simulink model "Complete_Model"  (R2023a)
settings: Solver=VariableStepAuto  StartTime=0.0  StopTime=22  MinStep=5e-5  RelTol=1e-3
values from neighbouring files (symbols this model uses):
  Cbus = 80e-3*n_wt_agg*u^2    (= 1.33333333333)    [param.m]
  D = 1e-3    (= 0.001)    [param.m]
  f = 50    [param.m]
  Fs = Vs * sqrt(2/3)/(2*pi*f)    [param.m]
  fsw = 4e3    (= 4000)    [param.m]
  Idr_rated = sqrt(2)*Is    [param.m]
  J = 127*n_wt_agg    (= 19050)    [param.m]
  ki_id = (wni^2)*Lr*sigma    (= 3.27733001332)    [param.m]
  ki_idg = (wnig^2)*Lg    (= 0.263189450696)    [param.m]
  ki_iq = ki_id    (= 3.27733001332)    [param.m]
  ki_iqg = ki_idg    (= 0.263189450696)    [param.m]
  ki_v = -300000*n_wt_agg    (= -45000000)    [param.m]
  Kopt = ((0.5*rho*pi*(Radius^5)*Cp_max)/(lambda_opt^3))    (= 296454.198186)    [param.m]
  kp_id = (2*wni*sigma*Lr) - Rr    (= 0.00384733333333)    [param.m]
  kp_idg = ((2*wnig*Lg)-Rg)    (= 0.00167538274858)    [param.m]
  kp_iq = kp_id    (= 0.00384733333333)    [param.m]
  kp_iqg = kp_idg    (= 0.00167538274858)    [param.m]
  kp_v = -1000*n_wt_agg    (= -150000)    [param.m]
  Kpg = 1/(1.5*Vr*sqrt(2/3))    [param.m]
  Kqg = -Kpg    [param.m]
  lambda_opt = 7.2    [param.m]
  Lg = 400e-6 /n_wt_agg    (= 2.66666666667e-06)    [param.m]
  Lm = 2.5e-3 /n_wt_agg    (= 1.66666666667e-05)    [param.m]
  Lr = Lm + Lsi    (= 1.72466666667e-05)    [param.m]
  Ls = Lm + Lsi    (= 1.72466666667e-05)    [param.m]
  Lsi = 0.087e-3 /n_wt_agg    (= 5.8e-07)    [param.m]
  N = 100    [param.m]
  n_wt_agg = 150    [param.m]
  p = 2    [param.m]
  Ps = 2e6*n_wt_agg    (= 300000000)    [param.m]
  Radius = 42    [param.m]
  Rg = 20e-6 /n_wt_agg    (= 1.33333333333e-07)    [param.m]
  rho = 1.225    [param.m]
  Rr = 2.9e-3 /n_wt_agg    (= 1.93333333333e-05)    [param.m]
  Rs = 2.6e-3 /n_wt_agg    (= 1.73333333333e-05)    [param.m]
  sigma = 1 - Lm^2 / (Ls*Lr)    (= 0.0661284179513)    [param.m]
  smax = 1/3    (= 0.333333333333)    [param.m]
  tab_lambda = [0.1:0.01:11.8]    [param.m]
  Vbus = 1150/u    (= 3450)    [param.m]
  Vs = 690    [param.m]
undefined symbols (not defined in the file or in its folder): Cp, D1, Delta, DiscIntNeverNeededParam, magnitude
libraries: spsSecondOrderFilterLib x133, spsThreePhaseVIMeasurementLib x58, spsPower3phInstantaneousLib x52, simulink x41, spsThreePhaseTransformerTwoWindingsLib x27, spsUniversalBridgeLib x22, spsPWMGenerator2LevelLib x22, spsSeriesRLCBranchLib x19, spsFirstOrderFilterLib x18, spsThreePhasePISectionLineLib x17, spsGroundLib x17, spsThreePhaseSeriesRLCBranchLib x17
(part 3 of 15)

== system Complete_Model/Equivalent Wind Park/300MW Eq WT3 ==
  66kV export cable 2 : spsThreePhasePISectionLineLib/Three-Phase PI Section Line  LConnTagsString=a|b|c  RConnTagsString=A|B|C  Length=10  Frequency=50  Resistances=[ 0.034/(n_wt_agg/35) 0.034/(n_wt_agg/35)]  Inductances="[ 0.34e-3/(n_wt_agg/35)   0.34e-3/(n_wt_agg/35) ]"  Capacitances="[0.29e-6*(n_wt_agg/35)  0.29e-6*(n_wt_agg/35) ]"
  Aerodynamic Model : Subsystem
  Asynchronous Machine SI Units : spsAsynchronousMachineSIUnitsLib/Asynchronous Machine SI Units  RotorType=Wound  PresetModel=No  MechanicalLoad=Torque Tm  ShowDetailedParameters=on  ReferenceFrame=Rotor  Units=SI  MeasurementBus=off  NominalParameters=[Ps Vs f]  VoltageRatio=1/u  Stator=[Rs Lsi]  Rotor=[Rr Lsi]  Cage1=[0.4155 0.002066]  Cage2=[0.4168 0.0003495]  Lm=Lm  Mechanical=[J D 2]  PolePairs=2  InitialConditions=[0.454 0 0 0 0 0 0 0]  SimulateSaturation=off  Saturation=[14.03593122, 27.81365428, 53.79336849, 72.68890987, 97.98006896, 148.6815601, 215.7428561, 302.9841135, 428.7778367 ; 230, 322, 414, 460, 506, 552, 598, 644, 690]  LoadFlowParameters=1492000  TsPowergui=0  TsBlock=-1  IterativeModel=Trapezoidal non iterative  IterativeDiscreteModel=Trapezoidal non iterative  Pmec=1.492e+006
  Constant3 : Constant  Value=Vbus
  DC_bus : spsSeriesRLCBranchLib/Series RLC Branch  LConnTagsString=__new0  RConnTagsString=__new0  BranchType=C  Resistance=1  Inductance=1e-3  SetiL0=off  InitialCurrent=0  Capacitance=Cbus  Setx0=on  InitialVoltage=Vbus  Measurements=Branch voltage
  Grid Measure : spsThreePhaseVIMeasurementLib/Three-Phase V-I Measurement  VoltageMeasurement=phase-to-ground  SetLabelV=on  LabelV=Vout10  Vpu=off  VpuLL=off  CurrentMeasurement=yes  SetLabelI=on  LabelI=Iout10  Ipu=off  Pbase=100e6  Vbase=500e3  OutputType=Complex  PhasorSimulation=off  PSBequivalent=0
  Grid Side Control : Subsystem
  Gridside_converter : spsUniversalBridgeLib/Universal Bridge  Arms=3  SnubberResistance=5000  SnubberCapacitance=1e-6  Device=Ideal Switches  Ron=1e-3  Lon=0  ForwardVoltages="[  0  0  ]"  ForwardVoltage=0  GTOparameters=[ 10e-6 , 20e-6 ]  IGBTparameters=[ 1e-6 , 2e-6 ]  Measurements=UAB UBC UCA UDC voltages  Measurements_2=None  converterType=Rectifier
  Ground2 : spsGroundLib/Ground  LConnTagsString=a
  PWM Generator (2-Level) : spsPWMGenerator2LevelLib/PWM Generator (2-Level)  ModulatorType=Three-phase bridge (6 pulses)  ModulatorMode=Unsynchronized  Fc=fsw  Pc=0  nF=27  MinMax="[ -1  1 ]"  SamplingTechnique=Symmetrical regular (single edge)  ModulatingSignals=off  m=0.8  Freq=60  Phase=0  Ts=0  ShowCarrierOutport=off
  PWM Generator (2-Level)1 : spsPWMGenerator2LevelLib/PWM Generator (2-Level)  ModulatorType=Three-phase bridge (6 pulses)  ModulatorMode=Unsynchronized  Fc=fsw  Pc=0  nF=27  MinMax="[ -1  1 ]"  SamplingTechnique=Symmetrical regular (single edge)  ModulatingSignals=off  m=0.8  Freq=60  Phase=0  Ts=0  ShowCarrierOutport=off
  Power Calculations : Subsystem
  Rate Limiter : RateLimiter  RisingSlewLimit=Ps/4  FallingSlewLimit=-Ps/4  SampleTimeMode=inherited
  Rotor Measure : spsThreePhaseVIMeasurementLib/Three-Phase V-I Measurement  VoltageMeasurement=phase-to-ground  SetLabelV=on  LabelV=Vr_mes10  Vpu=off  VpuLL=off  CurrentMeasurement=yes  SetLabelI=on  LabelI=Ir_mes10  Ipu=off  Pbase=100e6  Vbase=500e3  OutputType=Complex  PhasorSimulation=off  PSBequivalent=0
  Rotor Side Control : Subsystem
  Rotorside_converter : spsUniversalBridgeLib/Universal Bridge  Arms=3  SnubberResistance=5000  SnubberCapacitance=1e-6  Device=Ideal Switches  Ron=1e-3  Lon=0  ForwardVoltages="[  0  0  ]"  ForwardVoltage=0  GTOparameters=[ 10e-6 , 20e-6 ]  IGBTparameters=[ 1e-6 , 2e-6 ]  Measurements=None  Measurements_2=None  converterType=Rectifier
  Snubber Shunt : spsThreePhaseSeriesRLCBranchLib/Three-Phase Series RLC Branch  LConnTagsString=A|B|C  RConnTagsString=A|B|C  BranchType=R  Resistance=1e6  Inductance=Lg  Capacitance=1e-6  Measurements=None
  Stator Measure : spsThreePhaseVIMeasurementLib/Three-Phase V-I Measurement  VoltageMeasurement=phase-to-ground  SetLabelV=on  LabelV=Vs_mes_machine10  Vpu=off  VpuLL=off  CurrentMeasurement=yes  SetLabelI=on  LabelI=Is_mes_machine10  Ipu=off  Pbase=100e6  Vbase=500e3  OutputType=Complex  PhasorSimulation=off  PSBequivalent=0
  Subsystem : Subsystem
  Subsystem1 : Subsystem
  Subsystem2 : Subsystem
  Subsystem8 : Subsystem
  Three-Phase Transformer (Two Windings)1 : spsThreePhaseTransformerTwoWindingsLib/Three-Phase Transformer (Two Windings)  Winding1Connection=Yg  Winding2Connection=Yg  CoreType=Three single-phase transformers  SetSaturation=off  Hysteresis=off  DataFile='hysteresis'  SetInitialFlux=off  Measurements=None  UNITS=SI  NominalPower=[ Ps , f ]  Winding1=[690 0 0]  Winding2=[2070 Rg Lg]  Rm=500  Lm=500  L0=0.5  Saturation=[0 0;2840 2.152;1.1833e+06 2.7258]  InitialFluxes=[ 0.8 , -0.8 , 0.7 ]  BreakLoop=off  DiscreteSolver=Trapezoidal iterative  DataType=on  TransfoNumber=1
  Three-Phase Transformer (Two Windings)2 : spsThreePhaseTransformerTwoWindingsLib/Three-Phase Transformer (Two Windings)  Winding1Connection=Yg  Winding2Connection=Delta (D1)  CoreType=Three single-phase transformers  SetSaturation=off  Hysteresis=off  DataFile='hysteresis'  SetInitialFlux=off  Measurements=None  UNITS=pu  NominalPower=[ Ps , f ]  Winding1=[66e3 0.025/100 0.015]  Winding2=[Vs 0.025/100 0.015]  Rm=500  Lm=500  L0=0.5  Saturation=[0 0;0.22957 0.012546;95.654 0.015891]  InitialFluxes=[ 0.8 , -0.8 , 0.7 ]  BreakLoop=off  DiscreteSolver=Trapezoidal iterative  DataType=off  TransfoNumber=1
  Three-Phase V-I Measurement2 : spsThreePhaseVIMeasurementLib/Three-Phase V-I Measurement  VoltageMeasurement=phase-to-ground  SetLabelV=on  LabelV=Vg10  Vpu=off  VpuLL=off  CurrentMeasurement=yes  SetLabelI=on  LabelI=Ig10  Ipu=off  Pbase=100e6  Vbase=500e3  OutputType=Complex  PhasorSimulation=off  PSBequivalent=0
  Voltage Measurement : spsVoltageMeasurementLib/Voltage Measurement  PhasorSimulation=off  OutputType=Complex  PSBequivalent=0
  Zero-Order Hold{,1..8} (9 alike) : ZeroOrderHold  SampleTime=1/fsw
  big scope : Subsystem  Commented=on
  a : PMIOPort  Side=Left
  b : PMIOPort  Port=2  Side=Left
  c : PMIOPort  Port=3  Side=Left
  tag scope: Vbus_mes10=global
  wires:
  Asynchronous Machine SI Units/1 -> Subsystem/1(In1)
  Zero-Order Hold1/1 -> Rotor Side Control/4(theta_m_mes)
  Voltage Measurement/1 -> [Vbus_mes10]
  Subsystem1/1(Out1) -> Rate Limiter/1
  Zero-Order Hold5/1 -> Grid Side Control/1(Vbus_ref)
  Zero-Order Hold/1 -> Rotor Side Control/5(speed_mes)
  Zero-Order Hold2/1 -> Rotor Side Control/3(Vs_mes)
  [omega_m10] -> Zero-Order Hold/1
  Zero-Order Hold3/1 -> Rotor Side Control/2(Ir_mes)
  Zero-Order Hold6/1 -> Grid Side Control/5(Vs_mes)
  Zero-Order Hold7/1 -> Grid Side Control/4(Ig_mes)
  Zero-Order Hold8/1 -> Grid Side Control/3(Vbus)
  [Vout10] -> Zero-Order Hold6/1
  Rate Limiter/1 -> Zero-Order Hold4/1
  Subsystem2/1(v_wind) -> Aerodynamic Model/1(Vw)
  [Vout10] -> Zero-Order Hold2/1
  Zero-Order Hold4/1 -> Grid Side Control/2(Qg_ref)
  [Vbus_mes10] -> Zero-Order Hold8/1
  [Ig10] -> Zero-Order Hold7/1
  Constant3/1 -> Zero-Order Hold5/1
  Subsystem8/1(Out1) -> Rotor Side Control/1(idr_ref)
  [Ir_mes10] -> Zero-Order Hold3/1
  Aerodynamic Model/1(Tm) -> Asynchronous Machine SI Units/1
  Grid Side Control/1(Vabc_ref) -> PWM Generator (2-Level)1/1
  Rotor Side Control/1(Vabc_ref) -> PWM Generator (2-Level)/1
  [theta10] -> Zero-Order Hold1/1
  PWM Generator (2-Level)1/1 -> Gridside_converter/1
  PWM Generator (2-Level)/1 -> Rotorside_converter/1
  connections:
  Three-Phase Transformer (Two Windings)1/LConn1 -- Stator Measure/LConn1 -- Snubber Shunt/LConn1 -- Grid Measure/RConn1
  Asynchronous Machine SI Units/RConn2 -- Rotor Measure/RConn2
  Rotor Measure/LConn2 -- Rotorside_converter/LConn2
  Stator Measure/RConn2 -- Asynchronous Machine SI Units/LConn2
  Three-Phase Transformer (Two Windings)2/LConn3 -- 66kV export cable 2/RConn3
  66kV export cable 2/LConn3 -- c/RConn1
  Three-Phase Transformer (Two Windings)2/RConn2 -- Grid Measure/LConn2
  Three-Phase Transformer (Two Windings)2/RConn3 -- Grid Measure/LConn3
  Stator Measure/RConn1 -- Asynchronous Machine SI Units/LConn1
  Rotor Measure/LConn3 -- Rotorside_converter/LConn3
  Asynchronous Machine SI Units/RConn1 -- Rotor Measure/RConn1
  Three-Phase Transformer (Two Windings)2/LConn1 -- 66kV export cable 2/RConn1
  66kV export cable 2/LConn1 -- a/RConn1
  Asynchronous Machine SI Units/RConn3 -- Rotor Measure/RConn3
  Rotor Measure/LConn1 -- Rotorside_converter/LConn1
  Three-Phase V-I Measurement2/LConn1 -- Gridside_converter/LConn1
  Stator Measure/RConn3 -- Asynchronous Machine SI Units/LConn3
  Three-Phase Transformer (Two Windings)1/RConn1 -- Three-Phase V-I Measurement2/RConn1
  Three-Phase Transformer (Two Windings)1/RConn2 -- Three-Phase V-I Measurement2/RConn2
  Three-Phase V-I Measurement2/LConn3 -- Gridside_converter/LConn3
  Rotorside_converter/RConn1 -- DC_bus/LConn1 -- Gridside_converter/RConn1 -- Voltage Measurement/LConn1
  Three-Phase Transformer (Two Windings)2/LConn2 -- 66kV export cable 2/RConn2
  Three-Phase Transformer (Two Windings)2/RConn1 -- Grid Measure/LConn1
  Three-Phase Transformer (Two Windings)1/RConn3 -- Three-Phase V-I Measurement2/RConn3
  Snubber Shunt/LConn3 -- Grid Measure/RConn3 -- Three-Phase Transformer (Two Windings)1/LConn3 -- Stator Measure/LConn3
  Snubber Shunt/LConn2 -- Grid Measure/RConn2 -- Stator Measure/LConn2 -- Three-Phase Transformer (Two Windings)1/LConn2
  Snubber Shunt/RConn2 -- Snubber Shunt/RConn1 -- Snubber Shunt/RConn3 -- Ground2/LConn1
  Rotorside_converter/RConn2 -- Voltage Measurement/LConn2 -- Gridside_converter/RConn2 -- DC_bus/RConn1
  Three-Phase V-I Measurement2/LConn2 -- Gridside_converter/LConn2
  66kV export cable 2/LConn2 -- b/RConn1

== system Complete_Model/Equivalent Wind Park/300MW Eq WT4 ==
  66kV export cable 2 : spsThreePhasePISectionLineLib/Three-Phase PI Section Line  LConnTagsString=a|b|c  RConnTagsString=A|B|C  Length=10  Frequency=50  Resistances=[ 0.034/(n_wt_agg/35) 0.034/(n_wt_agg/35)]  Inductances="[ 0.34e-3/(n_wt_agg/35)   0.34e-3/(n_wt_agg/35) ]"  Capacitances="[0.29e-6*(n_wt_agg/35)  0.29e-6*(n_wt_agg/35) ]"
  Aerodynamic Model : Subsystem
  Asynchronous Machine SI Units : spsAsynchronousMachineSIUnitsLib/Asynchronous Machine SI Units  RotorType=Wound  PresetModel=No  MechanicalLoad=Torque Tm  ShowDetailedParameters=on  ReferenceFrame=Rotor  Units=SI  MeasurementBus=off  NominalParameters=[Ps Vs f]  VoltageRatio=1/u  Stator=[Rs Lsi]  Rotor=[Rr Lsi]  Cage1=[0.4155 0.002066]  Cage2=[0.4168 0.0003495]  Lm=Lm  Mechanical=[J D 2]  PolePairs=2  InitialConditions=[0.454 0 0 0 0 0 0 0]  SimulateSaturation=off  Saturation=[14.03593122, 27.81365428, 53.79336849, 72.68890987, 97.98006896, 148.6815601, 215.7428561, 302.9841135, 428.7778367 ; 230, 322, 414, 460, 506, 552, 598, 644, 690]  LoadFlowParameters=1492000  TsPowergui=0  TsBlock=-1  IterativeModel=Trapezoidal non iterative  IterativeDiscreteModel=Trapezoidal non iterative  Pmec=1.492e+006
  Constant3 : Constant  Value=Vbus
  DC_bus : spsSeriesRLCBranchLib/Series RLC Branch  LConnTagsString=__new0  RConnTagsString=__new0  BranchType=C  Resistance=1  Inductance=1e-3  SetiL0=off  InitialCurrent=0  Capacitance=Cbus  Setx0=on  InitialVoltage=Vbus  Measurements=Branch voltage
  Grid Measure : spsThreePhaseVIMeasurementLib/Three-Phase V-I Measurement  VoltageMeasurement=phase-to-ground  SetLabelV=on  LabelV=Vout9  Vpu=off  VpuLL=off  CurrentMeasurement=yes  SetLabelI=on  LabelI=Iout9  Ipu=off  Pbase=100e6  Vbase=500e3  OutputType=Complex  PhasorSimulation=off  PSBequivalent=0
  Grid Side Control : Subsystem
  Gridside_converter : spsUniversalBridgeLib/Universal Bridge  Arms=3  SnubberResistance=5000  SnubberCapacitance=1e-6  Device=Ideal Switches  Ron=1e-3  Lon=0  ForwardVoltages="[  0  0  ]"  ForwardVoltage=0  GTOparameters=[ 10e-6 , 20e-6 ]  IGBTparameters=[ 1e-6 , 2e-6 ]  Measurements=UAB UBC UCA UDC voltages  Measurements_2=None  converterType=Rectifier
  Ground2 : spsGroundLib/Ground  LConnTagsString=a
  PWM Generator (2-Level) : spsPWMGenerator2LevelLib/PWM Generator (2-Level)  ModulatorType=Three-phase bridge (6 pulses)  ModulatorMode=Unsynchronized  Fc=fsw  Pc=0  nF=27  MinMax="[ -1  1 ]"  SamplingTechnique=Symmetrical regular (single edge)  ModulatingSignals=off  m=0.8  Freq=60  Phase=0  Ts=0  ShowCarrierOutport=off
  PWM Generator (2-Level)1 : spsPWMGenerator2LevelLib/PWM Generator (2-Level)  ModulatorType=Three-phase bridge (6 pulses)  ModulatorMode=Unsynchronized  Fc=fsw  Pc=0  nF=27  MinMax="[ -1  1 ]"  SamplingTechnique=Symmetrical regular (single edge)  ModulatingSignals=off  m=0.8  Freq=60  Phase=0  Ts=0  ShowCarrierOutport=off
  Power Calculations : Subsystem
  Rate Limiter : RateLimiter  RisingSlewLimit=Ps/4  FallingSlewLimit=-Ps/4  SampleTimeMode=inherited
  Rotor Measure : spsThreePhaseVIMeasurementLib/Three-Phase V-I Measurement  VoltageMeasurement=phase-to-ground  SetLabelV=on  LabelV=Vr_mes9  Vpu=off  VpuLL=off  CurrentMeasurement=yes  SetLabelI=on  LabelI=Ir_mes9  Ipu=off  Pbase=100e6  Vbase=500e3  OutputType=Complex  PhasorSimulation=off  PSBequivalent=0
  Rotor Side Control : Subsystem
  Rotorside_converter : spsUniversalBridgeLib/Universal Bridge  Arms=3  SnubberResistance=5000  SnubberCapacitance=1e-6  Device=Ideal Switches  Ron=1e-3  Lon=0  ForwardVoltages="[  0  0  ]"  ForwardVoltage=0  GTOparameters=[ 10e-6 , 20e-6 ]  IGBTparameters=[ 1e-6 , 2e-6 ]  Measurements=None  Measurements_2=None  converterType=Rectifier
  Snubber Shunt : spsThreePhaseSeriesRLCBranchLib/Three-Phase Series RLC Branch  LConnTagsString=A|B|C  RConnTagsString=A|B|C  BranchType=R  Resistance=1e6  Inductance=Lg  Capacitance=1e-6  Measurements=None
  Stator Measure : spsThreePhaseVIMeasurementLib/Three-Phase V-I Measurement  VoltageMeasurement=phase-to-ground  SetLabelV=on  LabelV=Vs_mes_machine9  Vpu=off  VpuLL=off  CurrentMeasurement=yes  SetLabelI=on  LabelI=Is_mes_machine9  Ipu=off  Pbase=100e6  Vbase=500e3  OutputType=Complex  PhasorSimulation=off  PSBequivalent=0
  Subsystem : Subsystem
  Subsystem1 : Subsystem
  Subsystem2 : Subsystem
  Subsystem8 : Subsystem
  Three-Phase Transformer (Two Windings)1 : spsThreePhaseTransformerTwoWindingsLib/Three-Phase Transformer (Two Windings)  Winding1Connection=Yg  Winding2Connection=Yg  CoreType=Three single-phase transformers  SetSaturation=off  Hysteresis=off  DataFile='hysteresis'  SetInitialFlux=off  Measurements=None  UNITS=SI  NominalPower=[ Ps , f ]  Winding1=[690 0 0]  Winding2=[2070 Rg Lg]  Rm=500  Lm=500  L0=0.5  Saturation=[0 0;2840 2.152;1.1833e+06 2.7258]  InitialFluxes=[ 0.8 , -0.8 , 0.7 ]  BreakLoop=off  DiscreteSolver=Trapezoidal iterative  DataType=on  TransfoNumber=1
  Three-Phase Transformer (Two Windings)2 : spsThreePhaseTransformerTwoWindingsLib/Three-Phase Transformer (Two Windings)  Winding1Connection=Yg  Winding2Connection=Delta (D1)  CoreType=Three single-phase transformers  SetSaturation=off  Hysteresis=off  DataFile='hysteresis'  SetInitialFlux=off  Measurements=None  UNITS=pu  NominalPower=[ Ps , f ]  Winding1=[66e3 0.025/100 0.015]  Winding2=[Vs 0.025/100 0.015]  Rm=500  Lm=500  L0=0.5  Saturation=[0 0;0.22957 0.012546;95.654 0.015891]  InitialFluxes=[ 0.8 , -0.8 , 0.7 ]  BreakLoop=off  DiscreteSolver=Trapezoidal iterative  DataType=off  TransfoNumber=1
  Three-Phase V-I Measurement2 : spsThreePhaseVIMeasurementLib/Three-Phase V-I Measurement  VoltageMeasurement=phase-to-ground  SetLabelV=on  LabelV=Vg9  Vpu=off  VpuLL=off  CurrentMeasurement=yes  SetLabelI=on  LabelI=Ig9  Ipu=off  Pbase=100e6  Vbase=500e3  OutputType=Complex  PhasorSimulation=off  PSBequivalent=0
  Voltage Measurement : spsVoltageMeasurementLib/Voltage Measurement  PhasorSimulation=off  OutputType=Complex  PSBequivalent=0
  Zero-Order Hold{,1..8} (9 alike) : ZeroOrderHold  SampleTime=1/fsw
  big scope : Subsystem  Commented=on
  a : PMIOPort  Side=Left
  b : PMIOPort  Port=2  Side=Left
  c : PMIOPort  Port=3  Side=Left
  tag scope: Vbus_mes9=global
  wires:
  Asynchronous Machine SI Units/1 -> Subsystem/1(In1)
  Zero-Order Hold1/1 -> Rotor Side Control/4(theta_m_mes)
  Voltage Measurement/1 -> [Vbus_mes9]
  Subsystem1/1(Out1) -> Rate Limiter/1
  Zero-Order Hold5/1 -> Grid Side Control/1(Vbus_ref)
  Zero-Order Hold/1 -> Rotor Side Control/5(speed_mes)
  Zero-Order Hold2/1 -> Rotor Side Control/3(Vs_mes)
  [omega_m9] -> Zero-Order Hold/1
  Zero-Order Hold3/1 -> Rotor Side Control/2(Ir_mes)
  Zero-Order Hold6/1 -> Grid Side Control/5(Vs_mes)
  Zero-Order Hold7/1 -> Grid Side Control/4(Ig_mes)
  Zero-Order Hold8/1 -> Grid Side Control/3(Vbus)
  [Vout9] -> Zero-Order Hold6/1
  Rate Limiter/1 -> Zero-Order Hold4/1
  Subsystem2/1(v_wind) -> Aerodynamic Model/1(Vw)
  [Vout9] -> Zero-Order Hold2/1
  Zero-Order Hold4/1 -> Grid Side Control/2(Qg_ref)
  [Vbus_mes9] -> Zero-Order Hold8/1
  [Ig9] -> Zero-Order Hold7/1
  Constant3/1 -> Zero-Order Hold5/1
  Subsystem8/1(Out1) -> Rotor Side Control/1(idr_ref)
  [Ir_mes9] -> Zero-Order Hold3/1
  Aerodynamic Model/1(Tm) -> Asynchronous Machine SI Units/1
  Grid Side Control/1(Vabc_ref) -> PWM Generator (2-Level)1/1
  Rotor Side Control/1(Vabc_ref) -> PWM Generator (2-Level)/1
  [theta9] -> Zero-Order Hold1/1
  PWM Generator (2-Level)1/1 -> Gridside_converter/1
  PWM Generator (2-Level)/1 -> Rotorside_converter/1
  connections:
  Three-Phase Transformer (Two Windings)1/LConn1 -- Stator Measure/LConn1 -- Snubber Shunt/LConn1 -- Grid Measure/RConn1
  Asynchronous Machine SI Units/RConn2 -- Rotor Measure/RConn2
  Rotor Measure/LConn2 -- Rotorside_converter/LConn2
  Stator Measure/RConn2 -- Asynchronous Machine SI Units/LConn2
  Three-Phase Transformer (Two Windings)2/LConn3 -- 66kV export cable 2/RConn3
  66kV export cable 2/LConn3 -- c/RConn1
  Three-Phase Transformer (Two Windings)2/RConn2 -- Grid Measure/LConn2
  Three-Phase Transformer (Two Windings)2/RConn3 -- Grid Measure/LConn3
  Stator Measure/RConn1 -- Asynchronous Machine SI Units/LConn1
  Rotor Measure/LConn3 -- Rotorside_converter/LConn3
  Asynchronous Machine SI Units/RConn1 -- Rotor Measure/RConn1
  Three-Phase Transformer (Two Windings)2/LConn1 -- 66kV export cable 2/RConn1
  66kV export cable 2/LConn1 -- a/RConn1
  Asynchronous Machine SI Units/RConn3 -- Rotor Measure/RConn3
  Rotor Measure/LConn1 -- Rotorside_converter/LConn1
  Three-Phase V-I Measurement2/LConn1 -- Gridside_converter/LConn1
  Stator Measure/RConn3 -- Asynchronous Machine SI Units/LConn3
  Three-Phase Transformer (Two Windings)1/RConn1 -- Three-Phase V-I Measurement2/RConn1
  Three-Phase Transformer (Two Windings)1/RConn2 -- Three-Phase V-I Measurement2/RConn2
  Three-Phase V-I Measurement2/LConn3 -- Gridside_converter/LConn3
  Rotorside_converter/RConn1 -- DC_bus/LConn1 -- Gridside_converter/RConn1 -- Voltage Measurement/LConn1
  Three-Phase Transformer (Two Windings)2/LConn2 -- 66kV export cable 2/RConn2
  Three-Phase Transformer (Two Windings)2/RConn1 -- Grid Measure/LConn1
  Three-Phase Transformer (Two Windings)1/RConn3 -- Three-Phase V-I Measurement2/RConn3
  Snubber Shunt/LConn3 -- Grid Measure/RConn3 -- Three-Phase Transformer (Two Windings)1/LConn3 -- Stator Measure/LConn3
  Snubber Shunt/LConn2 -- Grid Measure/RConn2 -- Stator Measure/LConn2 -- Three-Phase Transformer (Two Windings)1/LConn2
  Snubber Shunt/RConn2 -- Snubber Shunt/RConn1 -- Snubber Shunt/RConn3 -- Ground2/LConn1
  Rotorside_converter/RConn2 -- Voltage Measurement/LConn2 -- Gridside_converter/RConn2 -- DC_bus/RConn1
  Three-Phase V-I Measurement2/LConn2 -- Gridside_converter/LConn2
  66kV export cable 2/LConn2 -- b/RConn1

== system Complete_Model/Equivalent Wind Park/300MW Eq WT5 ==
  66kV export cable 2 : spsThreePhasePISectionLineLib/Three-Phase PI Section Line  LConnTagsString=a|b|c  RConnTagsString=A|B|C  Length=10  Frequency=50  Resistances=[ 0.034/(n_wt_agg/35) 0.034/(n_wt_agg/35)]  Inductances="[ 0.34e-3/(n_wt_agg/35)   0.34e-3/(n_wt_agg/35) ]"  Capacitances="[0.29e-6*(n_wt_agg/35)  0.29e-6*(n_wt_agg/35) ]"
  Aerodynamic Model : Subsystem
  Asynchronous Machine SI Units : spsAsynchronousMachineSIUnitsLib/Asynchronous Machine SI Units  RotorType=Wound  PresetModel=No  MechanicalLoad=Torque Tm  ShowDetailedParameters=on  ReferenceFrame=Rotor  Units=SI  MeasurementBus=off  NominalParameters=[Ps Vs f]  VoltageRatio=1/u  Stator=[Rs Lsi]  Rotor=[Rr Lsi]  Cage1=[0.4155 0.002066]  Cage2=[0.4168 0.0003495]  Lm=Lm  Mechanical=[J D 2]  PolePairs=2  InitialConditions=[0.454 0 0 0 0 0 0 0]  SimulateSaturation=off  Saturation=[14.03593122, 27.81365428, 53.79336849, 72.68890987, 97.98006896, 148.6815601, 215.7428561, 302.9841135, 428.7778367 ; 230, 322, 414, 460, 506, 552, 598, 644, 690]  LoadFlowParameters=1492000  TsPowergui=0  TsBlock=-1  IterativeModel=Trapezoidal non iterative  IterativeDiscreteModel=Trapezoidal non iterative  Pmec=1.492e+006
  Constant3 : Constant  Value=Vbus
  DC_bus : spsSeriesRLCBranchLib/Series RLC Branch  LConnTagsString=__new0  RConnTagsString=__new0  BranchType=C  Resistance=1  Inductance=1e-3  SetiL0=off  InitialCurrent=0  Capacitance=Cbus  Setx0=on  InitialVoltage=Vbus  Measurements=Branch voltage
  Grid Measure : spsThreePhaseVIMeasurementLib/Three-Phase V-I Measurement  VoltageMeasurement=phase-to-ground  SetLabelV=on  LabelV=Vout5  Vpu=off  VpuLL=off  CurrentMeasurement=yes  SetLabelI=on  LabelI=Iout5  Ipu=off  Pbase=100e6  Vbase=500e3  OutputType=Complex  PhasorSimulation=off  PSBequivalent=0
  Grid Side Control : Subsystem
  Gridside_converter : spsUniversalBridgeLib/Universal Bridge  Arms=3  SnubberResistance=5000  SnubberCapacitance=1e-6  Device=Ideal Switches  Ron=1e-3  Lon=0  ForwardVoltages="[  0  0  ]"  ForwardVoltage=0  GTOparameters=[ 10e-6 , 20e-6 ]  IGBTparameters=[ 1e-6 , 2e-6 ]  Measurements=UAB UBC UCA UDC voltages  Measurements_2=None  converterType=Rectifier
  Ground2 : spsGroundLib/Ground  LConnTagsString=a
  PWM Generator (2-Level) : spsPWMGenerator2LevelLib/PWM Generator (2-Level)  ModulatorType=Three-phase bridge (6 pulses)  ModulatorMode=Unsynchronized  Fc=fsw  Pc=0  nF=27  MinMax="[ -1  1 ]"  SamplingTechnique=Symmetrical regular (single edge)  ModulatingSignals=off  m=0.8  Freq=60  Phase=0  Ts=0  ShowCarrierOutport=off
  PWM Generator (2-Level)1 : spsPWMGenerator2LevelLib/PWM Generator (2-Level)  ModulatorType=Three-phase bridge (6 pulses)  ModulatorMode=Unsynchronized  Fc=fsw  Pc=0  nF=27  MinMax="[ -1  1 ]"  SamplingTechnique=Symmetrical regular (single edge)  ModulatingSignals=off  m=0.8  Freq=60  Phase=0  Ts=0  ShowCarrierOutport=off
  Power Calculations : Subsystem
  Rate Limiter : RateLimiter  RisingSlewLimit=Ps/4  FallingSlewLimit=-Ps/4  SampleTimeMode=inherited
  Rotor Measure : spsThreePhaseVIMeasurementLib/Three-Phase V-I Measurement  VoltageMeasurement=phase-to-ground  SetLabelV=on  LabelV=Vr_mes5  Vpu=off  VpuLL=off  CurrentMeasurement=yes  SetLabelI=on  LabelI=Ir_mes5  Ipu=off  Pbase=100e6  Vbase=500e3  OutputType=Complex  PhasorSimulation=off  PSBequivalent=0
  Rotor Side Control : Subsystem
  Rotorside_converter : spsUniversalBridgeLib/Universal Bridge  Arms=3  SnubberResistance=5000  SnubberCapacitance=1e-6  Device=Ideal Switches  Ron=1e-3  Lon=0  ForwardVoltages="[  0  0  ]"  ForwardVoltage=0  GTOparameters=[ 10e-6 , 20e-6 ]  IGBTparameters=[ 1e-6 , 2e-6 ]  Measurements=None  Measurements_2=None  converterType=Rectifier
  Snubber Shunt : spsThreePhaseSeriesRLCBranchLib/Three-Phase Series RLC Branch  LConnTagsString=A|B|C  RConnTagsString=A|B|C  BranchType=R  Resistance=1e6  Inductance=Lg  Capacitance=1e-6  Measurements=None
  Stator Measure : spsThreePhaseVIMeasurementLib/Three-Phase V-I Measurement  VoltageMeasurement=phase-to-ground  SetLabelV=on  LabelV=Vs_mes_machine5  Vpu=off  VpuLL=off  CurrentMeasurement=yes  SetLabelI=on  LabelI=Is_mes_machine5  Ipu=off  Pbase=100e6  Vbase=500e3  OutputType=Complex  PhasorSimulation=off  PSBequivalent=0
  Subsystem : Subsystem
  Subsystem1 : Subsystem
  Subsystem2 : Subsystem
  Subsystem8 : Subsystem
  Three-Phase Transformer (Two Windings)1 : spsThreePhaseTransformerTwoWindingsLib/Three-Phase Transformer (Two Windings)  Winding1Connection=Yg  Winding2Connection=Yg  CoreType=Three single-phase transformers  SetSaturation=off  Hysteresis=off  DataFile='hysteresis'  SetInitialFlux=off  Measurements=None  UNITS=SI  NominalPower=[ Ps , f ]  Winding1=[690 0 0]  Winding2=[2070 Rg Lg]  Rm=500  Lm=500  L0=0.5  Saturation=[0 0;2840 2.152;1.1833e+06 2.7258]  InitialFluxes=[ 0.8 , -0.8 , 0.7 ]  BreakLoop=off  DiscreteSolver=Trapezoidal iterative  DataType=on  TransfoNumber=1
  Three-Phase Transformer (Two Windings)2 : spsThreePhaseTransformerTwoWindingsLib/Three-Phase Transformer (Two Windings)  Winding1Connection=Yg  Winding2Connection=Delta (D1)  CoreType=Three single-phase transformers  SetSaturation=off  Hysteresis=off  DataFile='hysteresis'  SetInitialFlux=off  Measurements=None  UNITS=pu  NominalPower=[ Ps , f ]  Winding1=[66e3 0.025/100 0.015]  Winding2=[Vs 0.025/100 0.015]  Rm=500  Lm=500  L0=0.5  Saturation=[0 0;0.22957 0.012546;95.654 0.015891]  InitialFluxes=[ 0.8 , -0.8 , 0.7 ]  BreakLoop=off  DiscreteSolver=Trapezoidal iterative  DataType=off  TransfoNumber=1
  Three-Phase V-I Measurement2 : spsThreePhaseVIMeasurementLib/Three-Phase V-I Measurement  VoltageMeasurement=phase-to-ground  SetLabelV=on  LabelV=Vg5  Vpu=off  VpuLL=off  CurrentMeasurement=yes  SetLabelI=on  LabelI=Ig5  Ipu=off  Pbase=100e6  Vbase=500e3  OutputType=Complex  PhasorSimulation=off  PSBequivalent=0
  Voltage Measurement : spsVoltageMeasurementLib/Voltage Measurement  PhasorSimulation=off  OutputType=Complex  PSBequivalent=0
  Zero-Order Hold{,1..8} (9 alike) : ZeroOrderHold  SampleTime=1/fsw
  big scope : Subsystem  Commented=on
  a : PMIOPort  Side=Left
  b : PMIOPort  Port=2  Side=Left
  c : PMIOPort  Port=3  Side=Left
  tag scope: Vbus_mes5=global
  wires:
  Asynchronous Machine SI Units/1 -> Subsystem/1(In1)
  Zero-Order Hold1/1 -> Rotor Side Control/4(theta_m_mes)
  Voltage Measurement/1 -> [Vbus_mes5]
  Subsystem1/1(Out1) -> Rate Limiter/1
  Zero-Order Hold5/1 -> Grid Side Control/1(Vbus_ref)
  Zero-Order Hold/1 -> Rotor Side Control/5(speed_mes)
  Zero-Order Hold2/1 -> Rotor Side Control/3(Vs_mes)
  [omega_m5] -> Zero-Order Hold/1
  Zero-Order Hold3/1 -> Rotor Side Control/2(Ir_mes)
  Zero-Order Hold6/1 -> Grid Side Control/5(Vs_mes)
  Zero-Order Hold7/1 -> Grid Side Control/4(Ig_mes)
  Zero-Order Hold8/1 -> Grid Side Control/3(Vbus)
  [Vout5] -> Zero-Order Hold6/1
  Rate Limiter/1 -> Zero-Order Hold4/1
  Subsystem2/1(v_wind) -> Aerodynamic Model/1(Vw)
  [Vout5] -> Zero-Order Hold2/1
  Zero-Order Hold4/1 -> Grid Side Control/2(Qg_ref)
  [Vbus_mes5] -> Zero-Order Hold8/1
  [Ig5] -> Zero-Order Hold7/1
  Constant3/1 -> Zero-Order Hold5/1
  Subsystem8/1(Out1) -> Rotor Side Control/1(idr_ref)
  [Ir_mes5] -> Zero-Order Hold3/1
  Aerodynamic Model/1(Tm) -> Asynchronous Machine SI Units/1
  Grid Side Control/1(Vabc_ref) -> PWM Generator (2-Level)1/1
  Rotor Side Control/1(Vabc_ref) -> PWM Generator (2-Level)/1
  [theta5] -> Zero-Order Hold1/1
  PWM Generator (2-Level)1/1 -> Gridside_converter/1
  PWM Generator (2-Level)/1 -> Rotorside_converter/1
  connections:
  Three-Phase Transformer (Two Windings)1/LConn1 -- Stator Measure/LConn1 -- Snubber Shunt/LConn1 -- Grid Measure/RConn1
  Asynchronous Machine SI Units/RConn2 -- Rotor Measure/RConn2
  Rotor Measure/LConn2 -- Rotorside_converter/LConn2
  Stator Measure/RConn2 -- Asynchronous Machine SI Units/LConn2
  Three-Phase Transformer (Two Windings)2/LConn3 -- 66kV export cable 2/RConn3
  66kV export cable 2/LConn3 -- c/RConn1
  Three-Phase Transformer (Two Windings)2/RConn2 -- Grid Measure/LConn2
  Three-Phase Transformer (Two Windings)2/RConn3 -- Grid Measure/LConn3
  Stator Measure/RConn1 -- Asynchronous Machine SI Units/LConn1
  Rotor Measure/LConn3 -- Rotorside_converter/LConn3
  Asynchronous Machine SI Units/RConn1 -- Rotor Measure/RConn1
  Three-Phase Transformer (Two Windings)2/LConn1 -- 66kV export cable 2/RConn1
  66kV export cable 2/LConn1 -- a/RConn1
  Asynchronous Machine SI Units/RConn3 -- Rotor Measure/RConn3
  Rotor Measure/LConn1 -- Rotorside_converter/LConn1
  Three-Phase V-I Measurement2/LConn1 -- Gridside_converter/LConn1
  Stator Measure/RConn3 -- Asynchronous Machine SI Units/LConn3
  Three-Phase Transformer (Two Windings)1/RConn1 -- Three-Phase V-I Measurement2/RConn1
  Three-Phase Transformer (Two Windings)1/RConn2 -- Three-Phase V-I Measurement2/RConn2
  Three-Phase V-I Measurement2/LConn3 -- Gridside_converter/LConn3
  Rotorside_converter/RConn1 -- DC_bus/LConn1 -- Gridside_converter/RConn1 -- Voltage Measurement/LConn1
  Three-Phase Transformer (Two Windings)2/LConn2 -- 66kV export cable 2/RConn2
  Three-Phase Transformer (Two Windings)2/RConn1 -- Grid Measure/LConn1
  Three-Phase Transformer (Two Windings)1/RConn3 -- Three-Phase V-I Measurement2/RConn3
  Snubber Shunt/LConn3 -- Grid Measure/RConn3 -- Three-Phase Transformer (Two Windings)1/LConn3 -- Stator Measure/LConn3
  Snubber Shunt/LConn2 -- Grid Measure/RConn2 -- Stator Measure/LConn2 -- Three-Phase Transformer (Two Windings)1/LConn2
  Snubber Shunt/RConn2 -- Snubber Shunt/RConn1 -- Snubber Shunt/RConn3 -- Ground2/LConn1
  Rotorside_converter/RConn2 -- Voltage Measurement/LConn2 -- Gridside_converter/RConn2 -- DC_bus/RConn1
  Three-Phase V-I Measurement2/LConn2 -- Gridside_converter/LConn2
  66kV export cable 2/LConn2 -- b/RConn1

== system Complete_Model/Equivalent Wind Park/300MW Eq WT6 ==
  66kV export cable 2 : spsThreePhasePISectionLineLib/Three-Phase PI Section Line  LConnTagsString=a|b|c  RConnTagsString=A|B|C  Length=10  Frequency=50  Resistances=[ 0.034/(n_wt_agg/35) 0.034/(n_wt_agg/35)]  Inductances="[ 0.34e-3/(n_wt_agg/35)   0.34e-3/(n_wt_agg/35) ]"  Capacitances="[0.29e-6*(n_wt_agg/35)  0.29e-6*(n_wt_agg/35) ]"
  Aerodynamic Model : Subsystem
  Asynchronous Machine SI Units : spsAsynchronousMachineSIUnitsLib/Asynchronous Machine SI Units  RotorType=Wound  PresetModel=No  MechanicalLoad=Torque Tm  ShowDetailedParameters=on  ReferenceFrame=Rotor  Units=SI  MeasurementBus=off  NominalParameters=[Ps Vs f]  VoltageRatio=1/u  Stator=[Rs Lsi]  Rotor=[Rr Lsi]  Cage1=[0.4155 0.002066]  Cage2=[0.4168 0.0003495]  Lm=Lm  Mechanical=[J D 2]  PolePairs=2  InitialConditions=[0.454 0 0 0 0 0 0 0]  SimulateSaturation=off  Saturation=[14.03593122, 27.81365428, 53.79336849, 72.68890987, 97.98006896, 148.6815601, 215.7428561, 302.9841135, 428.7778367 ; 230, 322, 414, 460, 506, 552, 598, 644, 690]  LoadFlowParameters=1492000  TsPowergui=0  TsBlock=-1  IterativeModel=Trapezoidal non iterative  IterativeDiscreteModel=Trapezoidal non iterative  Pmec=1.492e+006
  Constant3 : Constant  Value=Vbus
  DC_bus : spsSeriesRLCBranchLib/Series RLC Branch  LConnTagsString=__new0  RConnTagsString=__new0  BranchType=C  Resistance=1  Inductance=1e-3  SetiL0=off  InitialCurrent=0  Capacitance=Cbus  Setx0=on  InitialVoltage=Vbus  Measurements=Branch voltage
  Grid Measure : spsThreePhaseVIMeasurementLib/Three-Phase V-I Measurement  VoltageMeasurement=phase-to-ground  SetLabelV=on  LabelV=Vout8  Vpu=off  VpuLL=off  CurrentMeasurement=yes  SetLabelI=on  LabelI=Iout8  Ipu=off  Pbase=100e6  Vbase=500e3  OutputType=Complex  PhasorSimulation=off  PSBequivalent=0
  Grid Side Control : Subsystem
  Gridside_converter : spsUniversalBridgeLib/Universal Bridge  Arms=3  SnubberResistance=5000  SnubberCapacitance=1e-6  Device=Ideal Switches  Ron=1e-3  Lon=0  ForwardVoltages="[  0  0  ]"  ForwardVoltage=0  GTOparameters=[ 10e-6 , 20e-6 ]  IGBTparameters=[ 1e-6 , 2e-6 ]  Measurements=UAB UBC UCA UDC voltages  Measurements_2=None  converterType=Rectifier
  Ground2 : spsGroundLib/Ground  LConnTagsString=a
  PWM Generator (2-Level) : spsPWMGenerator2LevelLib/PWM Generator (2-Level)  ModulatorType=Three-phase bridge (6 pulses)  ModulatorMode=Unsynchronized  Fc=fsw  Pc=0  nF=27  MinMax="[ -1  1 ]"  SamplingTechnique=Symmetrical regular (single edge)  ModulatingSignals=off  m=0.8  Freq=60  Phase=0  Ts=0  ShowCarrierOutport=off
  PWM Generator (2-Level)1 : spsPWMGenerator2LevelLib/PWM Generator (2-Level)  ModulatorType=Three-phase bridge (6 pulses)  ModulatorMode=Unsynchronized  Fc=fsw  Pc=0  nF=27  MinMax="[ -1  1 ]"  SamplingTechnique=Symmetrical regular (single edge)  ModulatingSignals=off  m=0.8  Freq=60  Phase=0  Ts=0  ShowCarrierOutport=off
  Power Calculations : Subsystem
  Rate Limiter : RateLimiter  RisingSlewLimit=Ps/4  FallingSlewLimit=-Ps/4  SampleTimeMode=inherited
  Rotor Measure : spsThreePhaseVIMeasurementLib/Three-Phase V-I Measurement  VoltageMeasurement=phase-to-ground  SetLabelV=on  LabelV=Vr_mes8  Vpu=off  VpuLL=off  CurrentMeasurement=yes  SetLabelI=on  LabelI=Ir_mes8  Ipu=off  Pbase=100e6  Vbase=500e3  OutputType=Complex  PhasorSimulation=off  PSBequivalent=0
  Rotor Side Control : Subsystem
  Rotorside_converter : spsUniversalBridgeLib/Universal Bridge  Arms=3  SnubberResistance=5000  SnubberCapacitance=1e-6  Device=Ideal Switches  Ron=1e-3  Lon=0  ForwardVoltages="[  0  0  ]"  ForwardVoltage=0  GTOparameters=[ 10e-6 , 20e-6 ]  IGBTparameters=[ 1e-6 , 2e-6 ]  Measurements=None  Measurements_2=None  converterType=Rectifier
  Snubber Shunt : spsThreePhaseSeriesRLCBranchLib/Three-Phase Series RLC Branch  LConnTagsString=A|B|C  RConnTagsString=A|B|C  BranchType=R  Resistance=1e6  Inductance=Lg  Capacitance=1e-6  Measurements=None
  Stator Measure : spsThreePhaseVIMeasurementLib/Three-Phase V-I Measurement  VoltageMeasurement=phase-to-ground  SetLabelV=on  LabelV=Vs_mes_machine8  Vpu=off  VpuLL=off  CurrentMeasurement=yes  SetLabelI=on  LabelI=Is_mes_machine8  Ipu=off  Pbase=100e6  Vbase=500e3  OutputType=Complex  PhasorSimulation=off  PSBequivalent=0
  Subsystem : Subsystem
  Subsystem1 : Subsystem
  Subsystem2 : Subsystem
  Subsystem8 : Subsystem
  Three-Phase Transformer (Two Windings)1 : spsThreePhaseTransformerTwoWindingsLib/Three-Phase Transformer (Two Windings)  Winding1Connection=Yg  Winding2Connection=Yg  CoreType=Three single-phase transformers  SetSaturation=off  Hysteresis=off  DataFile='hysteresis'  SetInitialFlux=off  Measurements=None  UNITS=SI  NominalPower=[ Ps , f ]  Winding1=[690 0 0]  Winding2=[2070 Rg Lg]  Rm=500  Lm=500  L0=0.5  Saturation=[0 0;2840 2.152;1.1833e+06 2.7258]  InitialFluxes=[ 0.8 , -0.8 , 0.7 ]  BreakLoop=off  DiscreteSolver=Trapezoidal iterative  DataType=on  TransfoNumber=1
  Three-Phase Transformer (Two Windings)2 : spsThreePhaseTransformerTwoWindingsLib/Three-Phase Transformer (Two Windings)  Winding1Connection=Yg  Winding2Connection=Delta (D1)  CoreType=Three single-phase transformers  SetSaturation=off  Hysteresis=off  DataFile='hysteresis'  SetInitialFlux=off  Measurements=None  UNITS=pu  NominalPower=[ Ps , f ]  Winding1=[66e3 0.025/100 0.015]  Winding2=[Vs 0.025/100 0.015]  Rm=500  Lm=500  L0=0.5  Saturation=[0 0;0.22957 0.012546;95.654 0.015891]  InitialFluxes=[ 0.8 , -0.8 , 0.7 ]  BreakLoop=off  DiscreteSolver=Trapezoidal iterative  DataType=off  TransfoNumber=1
  Three-Phase V-I Measurement2 : spsThreePhaseVIMeasurementLib/Three-Phase V-I Measurement  VoltageMeasurement=phase-to-ground  SetLabelV=on  LabelV=Vg8  Vpu=off  VpuLL=off  CurrentMeasurement=yes  SetLabelI=on  LabelI=Ig8  Ipu=off  Pbase=100e6  Vbase=500e3  OutputType=Complex  PhasorSimulation=off  PSBequivalent=0
  Voltage Measurement : spsVoltageMeasurementLib/Voltage Measurement  PhasorSimulation=off  OutputType=Complex  PSBequivalent=0
  Zero-Order Hold{,1..8} (9 alike) : ZeroOrderHold  SampleTime=1/fsw
  big scope : Subsystem  Commented=on
  a : PMIOPort  Side=Left
  b : PMIOPort  Port=2  Side=Left
  c : PMIOPort  Port=3  Side=Left
  tag scope: Vbus_mes8=global
  wires:
  Asynchronous Machine SI Units/1 -> Subsystem/1(In1)
  Zero-Order Hold1/1 -> Rotor Side Control/4(theta_m_mes)
  Voltage Measurement/1 -> [Vbus_mes8]
  Subsystem1/1(Out1) -> Rate Limiter/1
  Zero-Order Hold5/1 -> Grid Side Control/1(Vbus_ref)
  Zero-Order Hold/1 -> Rotor Side Control/5(speed_mes)
  Zero-Order Hold2/1 -> Rotor Side Control/3(Vs_mes)
  [omega_m8] -> Zero-Order Hold/1
  Zero-Order Hold3/1 -> Rotor Side Control/2(Ir_mes)
  Zero-Order Hold6/1 -> Grid Side Control/5(Vs_mes)
  Zero-Order Hold7/1 -> Grid Side Control/4(Ig_mes)
  Zero-Order Hold8/1 -> Grid Side Control/3(Vbus)
  [Vout8] -> Zero-Order Hold6/1
  Rate Limiter/1 -> Zero-Order Hold4/1
  Subsystem2/1(v_wind) -> Aerodynamic Model/1(Vw)
  [Vout8] -> Zero-Order Hold2/1
  Zero-Order Hold4/1 -> Grid Side Control/2(Qg_ref)
  [Vbus_mes8] -> Zero-Order Hold8/1
  [Ig8] -> Zero-Order Hold7/1
  Constant3/1 -> Zero-Order Hold5/1
  Subsystem8/1(Out1) -> Rotor Side Control/1(idr_ref)
  [Ir_mes8] -> Zero-Order Hold3/1
  Aerodynamic Model/1(Tm) -> Asynchronous Machine SI Units/1
  Grid Side Control/1(Vabc_ref) -> PWM Generator (2-Level)1/1
  Rotor Side Control/1(Vabc_ref) -> PWM Generator (2-Level)/1
  [theta8] -> Zero-Order Hold1/1
  PWM Generator (2-Level)1/1 -> Gridside_converter/1
  PWM Generator (2-Level)/1 -> Rotorside_converter/1
  connections:
  Three-Phase Transformer (Two Windings)1/LConn1 -- Stator Measure/LConn1 -- Snubber Shunt/LConn1 -- Grid Measure/RConn1
  Asynchronous Machine SI Units/RConn2 -- Rotor Measure/RConn2
  Rotor Measure/LConn2 -- Rotorside_converter/LConn2
  Stator Measure/RConn2 -- Asynchronous Machine SI Units/LConn2
  Three-Phase Transformer (Two Windings)2/LConn3 -- 66kV export cable 2/RConn3
  66kV export cable 2/LConn3 -- c/RConn1
  Three-Phase Transformer (Two Windings)2/RConn2 -- Grid Measure/LConn2
  Three-Phase Transformer (Two Windings)2/RConn3 -- Grid Measure/LConn3
  Stator Measure/RConn1 -- Asynchronous Machine SI Units/LConn1
  Rotor Measure/LConn3 -- Rotorside_converter/LConn3
  Asynchronous Machine SI Units/RConn1 -- Rotor Measure/RConn1
  Three-Phase Transformer (Two Windings)2/LConn1 -- 66kV export cable 2/RConn1
  66kV export cable 2/LConn1 -- a/RConn1
  Asynchronous Machine SI Units/RConn3 -- Rotor Measure/RConn3
  Rotor Measure/LConn1 -- Rotorside_converter/LConn1
  Three-Phase V-I Measurement2/LConn1 -- Gridside_converter/LConn1
  Stator Measure/RConn3 -- Asynchronous Machine SI Units/LConn3
  Three-Phase Transformer (Two Windings)1/RConn1 -- Three-Phase V-I Measurement2/RConn1
  Three-Phase Transformer (Two Windings)1/RConn2 -- Three-Phase V-I Measurement2/RConn2
  Three-Phase V-I Measurement2/LConn3 -- Gridside_converter/LConn3
  Rotorside_converter/RConn1 -- DC_bus/LConn1 -- Gridside_converter/RConn1 -- Voltage Measurement/LConn1
  Three-Phase Transformer (Two Windings)2/LConn2 -- 66kV export cable 2/RConn2
  Three-Phase Transformer (Two Windings)2/RConn1 -- Grid Measure/LConn1
  Three-Phase Transformer (Two Windings)1/RConn3 -- Three-Phase V-I Measurement2/RConn3
  Snubber Shunt/LConn3 -- Grid Measure/RConn3 -- Three-Phase Transformer (Two Windings)1/LConn3 -- Stator Measure/LConn3
  Snubber Shunt/LConn2 -- Grid Measure/RConn2 -- Stator Measure/LConn2 -- Three-Phase Transformer (Two Windings)1/LConn2
  Snubber Shunt/RConn2 -- Snubber Shunt/RConn1 -- Snubber Shunt/RConn3 -- Ground2/LConn1
  Rotorside_converter/RConn2 -- Voltage Measurement/LConn2 -- Gridside_converter/RConn2 -- DC_bus/RConn1
  Three-Phase V-I Measurement2/LConn2 -- Gridside_converter/LConn2
  66kV export cable 2/LConn2 -- b/RConn1
